simulink library "lib_all"  (Simulink 7.6)
settings: Solver=ode45  SolverMode=SingleTasking  StartTime=0.0  StopTime=10.0  RelTol=1e-3
values from neighbouring files (symbols this model uses):
  map_lib_03 = [6.8679 7.0159 7.4571 8.1849 9.1892 10.456 11.97 13.71 15.655 17.781 20.063 22.474 ... (803 elements, 73x11)]    [scr_simdata.mat]    (also in scr_simdata_all.mat)
  map_lib_06 = [2.5784 2.6727 2.9588 3.4453 4.1444 5.068 6.226 7.6242 9.2634 11.139 13.24 15.552 ... (803 elements, 73x11)]    [scr_simdata.mat]    (also in scr_simdata_all.mat)
  map_lib_09 = [0.0025784 0.0026727 0.0029588 0.0034453 0.0041444 0.005068 0.006226 0.0076242 0.0092634 0.011139 0.01324 0.015552 ... (803 elements, 73x11)]    [scr_simdata.mat]    (also in scr_simdata_all.mat)
  map_lib_10 = [2.74 2.75 2.76 2.77 2.78 2.79 2.8 2.81 2.82 2.83 2.84 2.85 ... (81 elements, 1x81)]    [scr_simdata.mat]    (also in scr_simdata_all.mat)
  map_lib_11 = [1.1983 1.2027 1.207 1.2113 1.2156 1.2198 1.2241 1.2283 1.2326 1.2368 1.241 1.2451 ... (81 elements, 1x81)]    [scr_simdata.mat]    (also in scr_simdata_all.mat)
  map_lib_12 = [2.74 2.75 2.76 2.77 2.78 2.79 2.8 2.81 2.82 2.83 2.84 2.85 ... (81 elements, 1x81)]    [scr_simdata.mat]    (also in scr_simdata_all.mat)
  map_lib_13 = [1.74 1.75 1.76 1.77 1.78 1.79 1.8 1.81 1.82 1.83 1.84 1.85 ... (81 elements, 1x81)]    [scr_simdata.mat]    (also in scr_simdata_all.mat)
  map_lib_16 = [0.00048828125 1.6159 1.8962 2.0821 2.2251 2.3426 2.4433 2.5318 2.611 2.683 2.749 2.8101 ... (1419 elements, 129x11)]    [scr_simdata.mat]    (also in scr_simdata_all.mat)
  map_mr2_01 = [0 800 1600 2400 3200 4000]    [scr_simdata.mat]    (also in scr_simdata_all.mat)
  map_mr2_02 = [1.32 1.32 1.33 1.34 1.36 1.38]    [scr_simdata.mat]    (also in scr_simdata_all.mat)
  map_mr2_03 = [0 800 1600 2400 3200 4000]    [scr_simdata.mat]    (also in scr_simdata_all.mat)
  map_mr2_04 = [1.32 1.32 1.33 1.34 1.36 1.38]    [scr_simdata.mat]    (also in scr_simdata_all.mat)
  map_mr2_05 = [0 800 1600 2400 3200 4000]    [scr_simdata.mat]    (also in scr_simdata_all.mat)
  map_mr2_06 = [1.32 1.32 1.33 1.34 1.36 1.38]    [scr_simdata.mat]    (also in scr_simdata_all.mat)
  Parameter1 = 6    [scr_simdata.mat]    (also in scr_simdata_all.mat)
  Parameter101 = 30    [scr_simdata.mat]    (also in scr_simdata_all.mat)
  Parameter102 = 12    [scr_simdata.mat]    (also in scr_simdata_all.mat)
  Parameter103 = -240    [scr_simdata.mat]    (also in scr_simdata_all.mat)
  Parameter108 = 48    [scr_simdata.mat]    (also in scr_simdata_all.mat)
  Parameter120 = 16    [scr_simdata.mat]    (also in scr_simdata_all.mat)
  Parameter121 = 87    [scr_simdata.mat]    (also in scr_simdata_all.mat)
  Parameter123 = 78    [scr_simdata.mat]    (also in scr_simdata_all.mat)
  Parameter124 = 60    [scr_simdata.mat]    (also in scr_simdata_all.mat)
  Parameter125 = -480    [scr_simdata.mat]    (also in scr_simdata_all.mat)
  Parameter130 = 96    [scr_simdata.mat]    (also in scr_simdata_all.mat)
  Parameter131 = 3    [scr_simdata.mat]    (also in scr_simdata_all.mat)
  Parameter144 = 3    [scr_simdata.mat]    (also in scr_simdata_all.mat)
  Parameter18 = 128    [scr_simdata.mat]    (also in scr_simdata_all.mat)
  Parameter19 = 16    [scr_simdata.mat]    (also in scr_simdata_all.mat)
  Parameter2 = 12    [scr_simdata.mat]    (also in scr_simdata_all.mat)
  Parameter20 = 7    [scr_simdata.mat]    (also in scr_simdata_all.mat)
  Parameter21 = 4    [scr_simdata.mat]    (also in scr_simdata_all.mat)
  Parameter3 = 18    [scr_simdata.mat]    (also in scr_simdata_all.mat)
  Parameter32 = 1    [scr_simdata.mat]    (also in scr_simdata_all.mat)
  Parameter34 = 24    [scr_simdata.mat]    (also in scr_simdata_all.mat)
  Parameter4 = 138    [scr_simdata.mat]    (also in scr_simdata_all.mat)
  Parameter42 = 48    [scr_simdata.mat]    (also in scr_simdata_all.mat)
  Parameter5 = 135    [scr_simdata.mat]    (also in scr_simdata_all.mat)
  Parameter55 = 0    [scr_simdata.mat]    (also in scr_simdata_all.mat)
  Parameter56 = 0    [scr_simdata.mat]    (also in scr_simdata_all.mat)
  Parameter57 = 1    [scr_simdata.mat]    (also in scr_simdata_all.mat)
  Parameter58 = 1    [scr_simdata.mat]    (also in scr_simdata_all.mat)
  Parameter59 = 2    [scr_simdata.mat]    (also in scr_simdata_all.mat)
  Parameter6 = 132    [scr_simdata.mat]    (also in scr_simdata_all.mat)
  Parameter60 = 2    [scr_simdata.mat]    (also in scr_simdata_all.mat)
  Parameter63 = 144    [scr_simdata.mat]    (also in scr_simdata_all.mat)
  Parameter7 = 126    [scr_simdata.mat]    (also in scr_simdata_all.mat)
  Parameter76 = 64    [scr_simdata.mat]    (also in scr_simdata_all.mat)
  Parameter77 = 135    [scr_simdata.mat]    (also in scr_simdata_all.mat)
  Parameter79 = 126    [scr_simdata.mat]    (also in scr_simdata_all.mat)
  Parameter80 = 108    [scr_simdata.mat]    (also in scr_simdata_all.mat)
  Parameter81 = 0    [scr_simdata.mat]    (also in scr_simdata_all.mat)
  Parameter86 = 0    [scr_simdata.mat]    (also in scr_simdata_all.mat)
  Parameter98 = 112    [scr_simdata.mat]    (also in scr_simdata_all.mat)
  Parameter99 = 39    [scr_simdata.mat]    (also in scr_simdata_all.mat)
undefined symbols (not defined in the file or in its folder): cylvolkp_hih_map2h, cylvolkp_map2h, pk1k_map2h
libraries: simulink x2
(part 8 of 36)

== system lib_all/SubSystem1860/SubSystem1864/SubSystem1865 ==
  note: ***編集禁止***
  In1 : Inport
  In2 : Inport  Port=2
  LO1 : Logic
  RO1 : RelationalOperator  Operator=<  InputSameDT=on
  RO2 : RelationalOperator  Operator=~=  InputSameDT=on
  single1 : DataTypeConversion  OutDataTypeStr=single  SaturateOnIntegerOverflow=on  RndMeth=Zero
  single2 : DataTypeConversion  OutDataTypeStr=single  SaturateOnIntegerOverflow=on  RndMeth=Zero
  Out1 : Outport
  wires:
  In2/1 -> single2/1
  In1/1 -> single1/1
  single1/1 -> RO1/1, RO2/1
  single2/1 -> RO1/2, RO2/2
  RO1/1 -> LO1/1
  RO2/1 -> LO1/2
  LO1/1 -> Out1/1

== system lib_all/SubSystem1860/SubSystem1864/SubSystem1866 ==
  note: ***編集禁止***
  In1 : Inport
  In2 : Inport  Port=2
  RO1 : RelationalOperator  Operator===  InputSameDT=on
  single1 : DataTypeConversion  OutDataTypeStr=single  SaturateOnIntegerOverflow=on  RndMeth=Zero
  single2 : DataTypeConversion  OutDataTypeStr=single  SaturateOnIntegerOverflow=on  RndMeth=Zero
  Out1 : Outport
  wires:
  RO1/1 -> Out1/1
  In2/1 -> single2/1
  In1/1 -> single1/1
  single1/1 -> RO1/1
  single2/1 -> RO1/2

== system lib_all/SubSystem1860/SubSystem1864/SubSystem1867 ==
  Block5123 : Inport
  Block5124 : Inport  Port=2
  Block5125 : MultiPortSwitch  zeroidx=off  RndMeth=Zero  SaturateOnIntegerOverflow=on  InputSameDT=on
  SubSystem1868 : Subsystem  TreatAsAtomicUnit=on  mask(***ATC.DSRVEC***){bd_version=1.01.A, r0=DSM7, r1=-1, r2=, r3=, r4=, r5=, r6=, r7=, r8=, r9=, c0=, c1=, c2=, c3=, c4=, c5=, c6=, c7=, c8=, c9=}
      mask prompts: bd_version "Version"
      mask help: これはAutoCからのブロックです．編集禁止．
      settings: OpenFcn=warndlg('変更禁止!');
      mask Help: web(ui_blockhelpmng(gcb));
      mask Display: disp('ATC.DSRVEC')
  SubSystem1869 : Subsystem  TreatAsAtomicUnit=on  mask(***ATC.DSRVEC***){bd_version=1.01.A, r0=DSM6, r1=-1, r2=, r3=, r4=, r5=, r6=, r7=, r8=, r9=, c0=, c1=, c2=, c3=, c4=, c5=, c6=, c7=, c8=, c9=}
      mask prompts: bd_version "Version"
      mask help: これはAutoCからのブロックです．編集禁止．
      settings: OpenFcn=warndlg('変更禁止!');
      mask Help: web(ui_blockhelpmng(gcb));
      mask Display: disp('ATC.DSRVEC')
  SubSystem1870 : Subsystem  TreatAsAtomicUnit=on  mask(***ATC.DSRVEC***){bd_version=1.01.A, r0=DSM19, r1=-1, r2=, r3=, r4=, r5=, r6=, r7=, r8=, r9=, c0=, c1=, c2=, c3=, c4=, c5=, c6=, c7=, c8=, c9=}
      mask prompts: bd_version "Version"
      mask help: これはAutoCからのブロックです．編集禁止．
      settings: OpenFcn=warndlg('変更禁止!');
      mask Help: web(ui_blockhelpmng(gcb));
      mask Display: disp('ATC.DSRVEC')
  Block5126 : Outport
  wires:
  Block5123/1 -> Block5125/1
  Block5125/1 -> Block5126/1
  SubSystem1868/1(Output) -> Block5125/2
  SubSystem1869/1(Output) -> Block5125/3
  SubSystem1870/1(Output) -> Block5125/4
  Block5124/1 -> SubSystem1868/1(element), SubSystem1869/1(element), SubSystem1870/1(element)

== system lib_all/SubSystem1879/SubSystem1881/SubSystem1882 ==
  note: ***編集禁止***
  mult : Inport
  div : Inport  Port=2
  init : Inport  Port=3
  Product : Subsystem  TreatAsAtomicUnit=on
      settings: RTWSystemCode=Inline
  Out : Outport
  wires:
  Product/1(Out) -> Out/1
  mult/1 -> Product/1(mult)
  div/1 -> Product/2(div)
  init/1 -> Product/3(init)

== system lib_all/SubSystem1879/SubSystem1881/SubSystem1883 ==
  note: ***編集禁止***
  element : Inport
  Inside_DSR : DataStoreRead  DataStoreName=DSM95
  Selector : Selector  IndexOptions=Index vector (port)  Indices=[1 3]  OutputSizes=1
  Output : Outport
  wires:
  Selector/1 -> Output/1
  element/1 -> Selector/2
  Inside_DSR/1 -> Selector/1

== system lib_all/SubSystem1879/SubSystem1881/SubSystem1884 ==
  Block5162 : Inport
  Block5163 : Inport  Port=2
  Block5164 : Inport  Port=3
  Block5165 : Sum  OutDataTypeStr=double  RndMeth=Zero  SaturateOnIntegerOverflow=on  InputSameDT=on
  Block5166 : Sum  Inputs=+-  OutDataTypeStr=double  RndMeth=Zero  SaturateOnIntegerOverflow=on  InputSameDT=on
  Block5167 : Switch  Threshold=0.5  RndMeth=Zero  SaturateOnIntegerOverflow=on  InputSameDT=on
  Block5168 : Switch  Threshold=0.5  RndMeth=Zero  SaturateOnIntegerOverflow=on  InputSameDT=on
  SubSystem1885 : Subsystem
  SubSystem1886 : Subsystem
  Block5169 : Outport
  wires:
  Block5163/1 -> SubSystem1885/1(In1), Block5166/1, SubSystem1886/1(In1), Block5168/3, Block5165/2
  Block5162/1 -> Block5165/1, Block5166/2, SubSystem1885/2(In2)
  SubSystem1885/1(Out1) -> Block5167/2
  Block5164/1 -> SubSystem1886/2(In2)
  SubSystem1886/1(Out1) -> Block5168/2
  Block5168/1 -> Block5167/3
  Block5165/1 -> Block5168/1
  Block5167/1 -> Block5169/1
  Block5166/1 -> Block5167/1

== system lib_all/SubSystem1879/SubSystem1887/SubSystem1888 ==
  note: ***編集禁止***
  In1 : Inport
  In2 : Inport  Port=2
  LO1 : Logic
  RO1 : RelationalOperator  Operator=<  InputSameDT=on
  RO2 : RelationalOperator  Operator=~=  InputSameDT=on
  single1 : DataTypeConversion  OutDataTypeStr=single  SaturateOnIntegerOverflow=on  RndMeth=Zero
  single2 : DataTypeConversion  OutDataTypeStr=single  SaturateOnIntegerOverflow=on  RndMeth=Zero
  Out1 : Outport
  wires:
  LO1/1 -> Out1/1
  RO2/1 -> LO1/2
  RO1/1 -> LO1/1
  In2/1 -> single2/1
  In1/1 -> single1/1
  single1/1 -> RO2/1, RO1/1
  single2/1 -> RO2/2, RO1/2

== system lib_all/SubSystem1879/SubSystem1887/SubSystem1889 ==
  note: ***編集禁止***
  In1 : Inport
  In2 : Inport  Port=2
  RO1 : RelationalOperator  Operator===  InputSameDT=on
  single1 : DataTypeConversion  OutDataTypeStr=single  SaturateOnIntegerOverflow=on  RndMeth=Zero
  single2 : DataTypeConversion  OutDataTypeStr=single  SaturateOnIntegerOverflow=on  RndMeth=Zero
  Out1 : Outport
  wires:
  RO1/1 -> Out1/1
  In2/1 -> single2/1
  In1/1 -> single1/1
  single1/1 -> RO1/1
  single2/1 -> RO1/2

== system lib_all/SubSystem1879/SubSystem1887/SubSystem1890 ==
  note: ***編集禁止***
  In1 : Inport
  In2 : Inport  Port=2
  RO1 : RelationalOperator  Operator===  InputSameDT=on
  single1 : DataTypeConversion  OutDataTypeStr=single  SaturateOnIntegerOverflow=on  RndMeth=Zero
  single2 : DataTypeConversion  OutDataTypeStr=single  SaturateOnIntegerOverflow=on  RndMeth=Zero
  Out1 : Outport
  wires:
  RO1/1 -> Out1/1
  In2/1 -> single2/1
  In1/1 -> single1/1
  single1/1 -> RO1/1
  single2/1 -> RO1/2

== system lib_all/SubSystem1879/SubSystem1887/SubSystem1891 ==
  Block5190 : Inport
  Block5191 : Inport  Port=2
  Block5192 : EnablePort
  Block5193 : Demux  Outputs=2
  Block5194 : Mux  Inputs=2
  SubSystem1892 : Subsystem
  SubSystem1896 : Subsystem
  Block5203 : Outport
  wires:
  Block5190/1 -> Block5193/1
  Block5191/1 -> SubSystem1896/1(Block5199), SubSystem1892/1(Block5195)
  Block5193/1 -> SubSystem1892/2(Block5196)
  Block5193/2 -> SubSystem1896/2(Block5200)
  SubSystem1892/1(Block5198) -> Block5194/1
  SubSystem1896/1(Block5202) -> Block5194/2
  Block5194/1 -> Block5203/1

== system lib_all/SubSystem1879/SubSystem1887/SubSystem1900 ==
  Block5204 : Inport
  Block5205 : Inport  Port=2
  Block5206 : EnablePort
  Block5207 : Demux  Outputs=2
  Block5208 : Mux  Inputs=2
  Block5209 : Sum  OutDataTypeStr=double  RndMeth=Zero  SaturateOnIntegerOverflow=on  InputSameDT=on
  SubSystem1901 : Subsystem  TreatAsAtomicUnit=on  mask(***ATC.DSRVEC***){bd_version=1.01.A, r0=DSM4, r1=-1, r2=, r3=, r4=, r5=, r6=, r7=, r8=, r9=, c0=, c1=, c2=, c3=, c4=, c5=, c6=, c7=, c8=, c9=}
      mask prompts: bd_version "Version"
      mask help: これはAutoCからのブロックです．編集禁止．
      settings: OpenFcn=warndlg('変更禁止!');
      mask Help: web(ui_blockhelpmng(gcb));
      mask Display: disp('ATC.DSRVEC')
  SubSystem1902 : Subsystem
  SubSystem1906 : Subsystem
  Block5218 : Outport
  wires:
  Block5207/2 -> SubSystem1906/2(Block5215)
  Block5207/1 -> SubSystem1902/2(Block5211)
  Block5204/1 -> Block5207/1
  SubSystem1902/1(Block5213) -> Block5208/1
  SubSystem1906/1(Block5217) -> Block5208/2
  Block5209/1 -> Block5218/1
  SubSystem1901/1(Output) -> Block5209/1
  Block5208/1 -> Block5209/2
  Block5205/1 -> SubSystem1906/1(Block5214), SubSystem1902/1(Block5210), SubSystem1901/1(element)

== system lib_all/SubSystem1879/SubSystem1887/SubSystem1910 ==
  Block5219 : Inport
  Block5220 : Inport  Port=2
  Block5221 : EnablePort
  Block5222 : Demux  Outputs=2
  Block5223 : Mux  Inputs=2
  Block5224 : Sum  OutDataTypeStr=double  RndMeth=Zero  SaturateOnIntegerOverflow=on  InputSameDT=on
  SubSystem1911 : Subsystem  TreatAsAtomicUnit=on  mask(***ATC.DSRVEC***){bd_version=1.01.A, r0=DSM2, r1=-1, r2=, r3=, r4=, r5=, r6=, r7=, r8=, r9=, c0=, c1=, c2=, c3=, c4=, c5=, c6=, c7=, c8=, c9=}
      mask prompts: bd_version "Version"
      mask help: これはAutoCからのブロックです．編集禁止．
      settings: OpenFcn=warndlg('変更禁止!');
      mask Help: web(ui_blockhelpmng(gcb));
      mask Display: disp('ATC.DSRVEC')
  SubSystem1912 : Subsystem
  SubSystem1916 : Subsystem
  Block5233 : Outport
  wires:
  Block5219/1 -> Block5222/1
  Block5222/1 -> SubSystem1912/2(Block5226)
  Block5222/2 -> SubSystem1916/2(Block5230)
  SubSystem1912/1(Block5228) -> Block5223/1
  SubSystem1916/1(Block5232) -> Block5223/2
  Block5224/1 -> Block5233/1
  Block5223/1 -> Block5224/2
  Block5220/1 -> SubSystem1916/1(Block5229), SubSystem1912/1(Block5225), SubSystem1911/1(element)
  SubSystem1911/1(Output) -> Block5224/1

== system lib_all/SubSystem1920/SubSystem1922/SubSystem1923 ==
  note: ***編集禁止***
  mult : Inport
  div : Inport  Port=2
  init : Inport  Port=3
  Product : Subsystem  TreatAsAtomicUnit=on
      settings: RTWSystemCode=Inline
  Out : Outport
  wires:
  init/1 -> Product/3(init)
  div/1 -> Product/2(div)
  mult/1 -> Product/1(mult)
  Product/1(Out) -> Out/1

== system lib_all/SubSystem1920/SubSystem1922/SubSystem1924 ==
  note: ***編集禁止***
  element : Inport
  Inside_DSR : DataStoreRead  DataStoreName=DSM95
  Selector : Selector  IndexOptions=Index vector (port)  Indices=[1 3]  OutputSizes=1
  Output : Outport
  wires:
  Inside_DSR/1 -> Selector/1
  element/1 -> Selector/2
  Selector/1 -> Output/1

== system lib_all/SubSystem1920/SubSystem1922/SubSystem1925 ==
  Block5262 : Inport
  Block5263 : Inport  Port=2
  Block5264 : Inport  Port=3
  Block5265 : Sum  OutDataTypeStr=double  RndMeth=Zero  SaturateOnIntegerOverflow=on  InputSameDT=on
  Block5266 : Sum  Inputs=+-  OutDataTypeStr=double  RndMeth=Zero  SaturateOnIntegerOverflow=on  InputSameDT=on
  Block5267 : Switch  Threshold=0.5  RndMeth=Zero  SaturateOnIntegerOverflow=on  InputSameDT=on
  Block5268 : Switch  Threshold=0.5  RndMeth=Zero  SaturateOnIntegerOverflow=on  InputSameDT=on
  SubSystem1926 : Subsystem
  SubSystem1927 : Subsystem
  Block5269 : Outport
  wires:
  Block5266/1 -> Block5267/1
  Block5267/1 -> Block5269/1
  Block5265/1 -> Block5268/1
  Block5268/1 -> Block5267/3
  SubSystem1927/1(Out1) -> Block5268/2
  Block5264/1 -> SubSystem1927/2(In2)
  SubSystem1926/1(Out1) -> Block5267/2
  Block5262/1 -> SubSystem1926/2(In2), Block5266/2, Block5265/1
  Block5263/1 -> Block5265/2, Block5268/3, SubSystem1927/1(In1), Block5266/1, SubSystem1926/1(In1)

== system lib_all/SubSystem1920/SubSystem1928/SubSystem1929 ==
  note: ***編集禁止***
  In1 : Inport
  In2 : Inport  Port=2
  LO1 : Logic
  RO1 : RelationalOperator  Operator=<  InputSameDT=on
  RO2 : RelationalOperator  Operator=~=  InputSameDT=on
  single1 : DataTypeConversion  OutDataTypeStr=single  SaturateOnIntegerOverflow=on  RndMeth=Zero
  single2 : DataTypeConversion  OutDataTypeStr=single  SaturateOnIntegerOverflow=on  RndMeth=Zero
  Out1 : Outport
  wires:
  single2/1 -> RO1/2, RO2/2
  single1/1 -> RO1/1, RO2/1
  In1/1 -> single1/1
  In2/1 -> single2/1
  RO1/1 -> LO1/1
  RO2/1 -> LO1/2
  LO1/1 -> Out1/1

== system lib_all/SubSystem1920/SubSystem1928/SubSystem1930 ==
  note: ***編集禁止***
  In1 : Inport
  In2 : Inport  Port=2
  RO1 : RelationalOperator  Operator===  InputSameDT=on
  single1 : DataTypeConversion  OutDataTypeStr=single  SaturateOnIntegerOverflow=on  RndMeth=Zero
  single2 : DataTypeConversion  OutDataTypeStr=single  SaturateOnIntegerOverflow=on  RndMeth=Zero
  Out1 : Outport
  wires:
  single2/1 -> RO1/2
  single1/1 -> RO1/1
  In1/1 -> single1/1
  In2/1 -> single2/1
  RO1/1 -> Out1/1

== system lib_all/SubSystem1920/SubSystem1928/SubSystem1931 ==
  note: ***編集禁止***
  In1 : Inport
  In2 : Inport  Port=2
  RO1 : RelationalOperator  Operator===  InputSameDT=on
  single1 : DataTypeConversion  OutDataTypeStr=single  SaturateOnIntegerOverflow=on  RndMeth=Zero
  single2 : DataTypeConversion  OutDataTypeStr=single  SaturateOnIntegerOverflow=on  RndMeth=Zero
  Out1 : Outport
  wires:
  single2/1 -> RO1/2
  single1/1 -> RO1/1
  In1/1 -> single1/1
  In2/1 -> single2/1
  RO1/1 -> Out1/1

== system lib_all/SubSystem1920/SubSystem1928/SubSystem1932 ==
  Block5290 : Inport
  Block5291 : Inport  Port=2
  Block5292 : EnablePort
  Block5293 : Demux  Outputs=2
  Block5294 : Mux  Inputs=2
  SubSystem1933 : Subsystem
  SubSystem1937 : Subsystem
  Block5303 : Outport
  wires:
  Block5294/1 -> Block5303/1
  SubSystem1937/1(Block5302) -> Block5294/2
  SubSystem1933/1(Block5298) -> Block5294/1
  Block5293/2 -> SubSystem1937/2(Block5300)
  Block5293/1 -> SubSystem1933/2(Block5296)
  Block5291/1 -> SubSystem1933/1(Block5295), SubSystem1937/1(Block5299)
  Block5290/1 -> Block5293/1

== system lib_all/SubSystem1920/SubSystem1928/SubSystem1941 ==
  Block5304 : Inport
  Block5305 : Inport  Port=2
  Block5306 : EnablePort
  Block5307 : Demux  Outputs=2
  Block5308 : Mux  Inputs=2
  Block5309 : Sum  OutDataTypeStr=double  RndMeth=Zero  SaturateOnIntegerOverflow=on  InputSameDT=on
  SubSystem1942 : Subsystem  TreatAsAtomicUnit=on  mask(***ATC.DSRVEC***){bd_version=1.01.A, r0=DSM4, r1=-1, r2=, r3=, r4=, r5=, r6=, r7=, r8=, r9=, c0=, c1=, c2=, c3=, c4=, c5=, c6=, c7=, c8=, c9=}
      mask prompts: bd_version "Version"
      mask help: これはAutoCからのブロックです．編集禁止．
      settings: OpenFcn=warndlg('変更禁止!');
      mask Help: web(ui_blockhelpmng(gcb));
      mask Display: disp('ATC.DSRVEC')
  SubSystem1943 : Subsystem
  SubSystem1947 : Subsystem
  Block5318 : Outport
  wires:
  Block5305/1 -> SubSystem1942/1(element), SubSystem1943/1(Block5310), SubSystem1947/1(Block5314)
  Block5308/1 -> Block5309/2
  SubSystem1942/1(Output) -> Block5309/1
  Block5309/1 -> Block5318/1
  SubSystem1947/1(Block5317) -> Block5308/2
  SubSystem1943/1(Block5313) -> Block5308/1
  Block5304/1 -> Block5307/1
  Block5307/1 -> SubSystem1943/2(Block5311)
  Block5307/2 -> SubSystem1947/2(Block5315)

== system lib_all/SubSystem1920/SubSystem1928/SubSystem1951 ==
  Block5319 : Inport
  Block5320 : Inport  Port=2
  Block5321 : EnablePort
  Block5322 : Demux  Outputs=2
  Block5323 : Mux  Inputs=2
  Block5324 : Sum  OutDataTypeStr=double  RndMeth=Zero  SaturateOnIntegerOverflow=on  InputSameDT=on
  SubSystem1952 : Subsystem  TreatAsAtomicUnit=on  mask(***ATC.DSRVEC***){bd_version=1.01.A, r0=DSM2, r1=-1, r2=, r3=, r4=, r5=, r6=, r7=, r8=, r9=, c0=, c1=, c2=, c3=, c4=, c5=, c6=, c7=, c8=, c9=}
      mask prompts: bd_version "Version"
      mask help: これはAutoCからのブロックです．編集禁止．
      settings: OpenFcn=warndlg('変更禁止!');
      mask Help: web(ui_blockhelpmng(gcb));
      mask Display: disp('ATC.DSRVEC')
  SubSystem1953 : Subsystem
  SubSystem1957 : Subsystem
  Block5333 : Outport
  wires:
  SubSystem1952/1(Output) -> Block5324/1
  Block5320/1 -> SubSystem1952/1(element), SubSystem1953/1(Block5325), SubSystem1957/1(Block5329)
  Block5323/1 -> Block5324/2
  Block5324/1 -> Block5333/1
  SubSystem1957/1(Block5332) -> Block5323/2
  SubSystem1953/1(Block5328) -> Block5323/1
  Block5322/2 -> SubSystem1957/2(Block5330)
  Block5322/1 -> SubSystem1953/2(Block5326)
  Block5319/1 -> Block5322/1

== system lib_all/SubSystem347/SubSystem349/SubSystem350 ==
  note: ***編集禁止***
  In1 : Inport
  In2 : Inport  Port=2
  LO1 : Logic
  RO1 : RelationalOperator  Operator=<  InputSameDT=on
  RO2 : RelationalOperator  Operator=~=  InputSameDT=on
  single1 : DataTypeConversion  OutDataTypeStr=single  SaturateOnIntegerOverflow=on  RndMeth=Zero
  single2 : DataTypeConversion  OutDataTypeStr=single  SaturateOnIntegerOverflow=on  RndMeth=Zero
  Out1 : Outport
  wires:
  In2/1 -> single2/1
  In1/1 -> single1/1
  single1/1 -> RO1/1, RO2/1
  single2/1 -> RO1/2, RO2/2
  RO1/1 -> LO1/1
  RO2/1 -> LO1/2
  LO1/1 -> Out1/1

== system lib_all/SubSystem347/SubSystem349/SubSystem351 ==
  note: ***編集禁止***
  element : Inport
  Inside_DSR : DataStoreRead  DataStoreName=DSM386
  Selector : Selector  IndexOptions=Index vector (port)  Indices=[1 3]  OutputSizes=1
  Output : Outport
  wires:
  Selector/1 -> Output/1
  element/1 -> Selector/2
  Inside_DSR/1 -> Selector/1

== system lib_all/SubSystem347/SubSystem349/SubSystem352 ==
  Block767 : Inport
  Block768 : Inport  Port=2
  Block769 : Inport  Port=3
  Block770 : Inport  Port=4
  Block771 : EnablePort
  Block772 : Constant  OutDataTypeStr=double  SampleTime=-1
  Block773 : Constant  Value=0  OutDataTypeStr=double  SampleTime=-1
  Block774 : Constant  Value=0  OutDataTypeStr=double  SampleTime=-1
  Block775 : Sum  OutDataTypeStr=double  RndMeth=Zero  SaturateOnIntegerOverflow=on  InputSameDT=on
  SubSystem353 : Subsystem  TreatAsAtomicUnit=on
      settings: AttributesFormatString=Attribute171  RTWFcnNameOpts=Use subsystem name
  SubSystem406 : Subsystem  TreatAsAtomicUnit=on
      settings: AttributesFormatString=Attribute190  RTWFcnNameOpts=Use subsystem name
  SubSystem459 : Subsystem
  SubSystem466 : Subsystem
  SubSystem473 : Subsystem  Priority=3  TreatAsAtomicUnit=on
      settings: AttributesFormatString=Attribute209  RTWSystemCode=Inline
  wires:
  Block772/1 -> Block775/2
  Block767/1 -> SubSystem406/1(Block898), SubSystem353/1(Block776), SubSystem473/1(Block1048), Block775/1
  Block769/1 -> SubSystem353/2(Block777)
  Block768/1 -> SubSystem406/2(Block899)
  Block774/1 -> SubSystem353/4(Block779), SubSystem353/3(Block778)
  Block773/1 -> SubSystem406/4(Block901), SubSystem406/3(Block900)
  SubSystem353/1(Block897) -> SubSystem466/1(Block1034)
  Block770/1 -> SubSystem466/3(Block1036), SubSystem459/3(Block1022)
  Block775/1 -> SubSystem466/2(Block1035), SubSystem459/2(Block1021)
  SubSystem406/1(Block1019) -> SubSystem459/1(Block1020)

== system lib_all/SubSystem347/SubSystem349/SubSystem476 ==
  Block1053 : Inport
  Block1054 : Constant  Value=Parameter99  OutDataTypeStr=double  SampleTime=-1
  Block1055 : Constant  Value=Parameter121  OutDataTypeStr=double  SampleTime=-1
  Block1056 : Constant  Value=Parameter79  OutDataTypeStr=double  SampleTime=-1
  Block1057 : Constant  Value=Parameter77  OutDataTypeStr=double  SampleTime=-1
  Block1058 : Constant  Value=Parameter101  OutDataTypeStr=double  SampleTime=-1
  Block1059 : Constant  Value=Parameter123  OutDataTypeStr=double  SampleTime=-1
  Block1060 : MultiPortSwitch  zeroidx=off  RndMeth=Zero  SaturateOnIntegerOverflow=on  InputSameDT=on
  Block1061 : Mux  Inputs=2
  Block1062 : Mux  Inputs=2
  Block1063 : Mux  Inputs=2
  Block1064 : Outport
  wires:
  Block1056/1 -> Block1061/2
  Block1058/1 -> Block1062/2
  Block1059/1 -> Block1063/2
  Block1055/1 -> Block1063/1
  Block1054/1 -> Block1062/1
  Block1060/1 -> Block1064/1
  Block1053/1 -> Block1060/1
  Block1057/1 -> Block1061/1
  Block1061/1 -> Block1060/2
  Block1063/1 -> Block1060/4
  Block1062/1 -> Block1060/3

== system lib_all/SubSystem347/SubSystem477/SubSystem478 ==
  note: ***編集禁止***
  In1 : Inport
  In2 : Inport  Port=2
  RO1 : RelationalOperator  Operator===  InputSameDT=on
  single1 : DataTypeConversion  OutDataTypeStr=single  SaturateOnIntegerOverflow=on  RndMeth=Zero
  single2 : DataTypeConversion  OutDataTypeStr=single  SaturateOnIntegerOverflow=on  RndMeth=Zero
  Out1 : Outport
  wires:
  RO1/1 -> Out1/1
  In2/1 -> single2/1
  In1/1 -> single1/1
  single1/1 -> RO1/1
  single2/1 -> RO1/2

== system lib_all/SubSystem347/SubSystem477/SubSystem479 ==
  Block1069 : Inport
  Block1070 : EnablePort
  Block1071 : Constant  Value=Parameter18  OutDataTypeStr=double  SampleTime=-1
  SubSystem480 : Subsystem  TreatAsAtomicUnit=on
      settings: RTWSystemCode=Inline
  SubSystem482 : Subsystem
  SubSystem492 : Subsystem  TreatAsAtomicUnit=on
  wires:
  Block1069/1 -> SubSystem492/2(Block1096), SubSystem482/3(Block1077)
  Block1071/1 -> SubSystem480/1(N)
  SubSystem480/1(action) -> SubSystem492/Trigger
  SubSystem480/2(i) -> SubSystem492/1(Block1095)
  SubSystem492/1(Block1113) -> SubSystem482/1(Block1075)
  SubSystem492/2(Block1114) -> SubSystem482/2(Block1076)

== system lib_all/SubSystem347/SubSystem477/SubSystem502 ==
  note: ***編集禁止***
  element : Inport
  Inside_DSR : DataStoreRead  DataStoreName=DSM386
  Selector : Selector  IndexOptions=Index vector (port)  Indices=[1 3]  OutputSizes=1
  Output : Outport
  wires:
  Selector/1 -> Output/1
  element/1 -> Selector/2
  Inside_DSR/1 -> Selector/1

== system lib_all/SubSystem347/SubSystem503/SubSystem504 ==
  note: ***編集禁止***
  In1 : Inport
  In2 : Inport  Port=2
  LO1 : Logic  Operator=OR
  RO1 : RelationalOperator  InputSameDT=on
  RO2 : RelationalOperator  Operator===  InputSameDT=on
  single1 : DataTypeConversion  OutDataTypeStr=single  SaturateOnIntegerOverflow=on  RndMeth=Zero
  single2 : DataTypeConversion  OutDataTypeStr=single  SaturateOnIntegerOverflow=on  RndMeth=Zero
  Out1 : Outport
  wires:
  In2/1 -> single2/1
  In1/1 -> single1/1
  single1/1 -> RO1/1, RO2/1
  single2/1 -> RO1/2, RO2/2
  RO1/1 -> LO1/1
  RO2/1 -> LO1/2
  LO1/1 -> Out1/1

== system lib_all/SubSystem347/SubSystem503/SubSystem505 ==
  note: ***編集禁止***
  In1 : Inport
  In2 : Inport  Port=2
  RO1 : RelationalOperator  Operator===  InputSameDT=on
  single1 : DataTypeConversion  OutDataTypeStr=single  SaturateOnIntegerOverflow=on  RndMeth=Zero
  single2 : DataTypeConversion  OutDataTypeStr=single  SaturateOnIntegerOverflow=on  RndMeth=Zero
  Out1 : Outport
  wires:
  RO1/1 -> Out1/1
  In2/1 -> single2/1
  In1/1 -> single1/1
  single1/1 -> RO1/1
  single2/1 -> RO1/2

== system lib_all/SubSystem347/SubSystem503/SubSystem506 ==
  Block1119 : EnablePort
  Block1120 : Constant  Value=Parameter131  OutDataTypeStr=double  SampleTime=-1
  SubSystem507 : Subsystem  TreatAsAtomicUnit=on
      settings: RTWSystemCode=Inline
  SubSystem509 : Subsystem  TreatAsAtomicUnit=on
      settings: RTWSystemCode=Inline
  SubSystem511 : Subsystem  TreatAsAtomicUnit=on
  SubSystem515 : Subsystem  TreatAsAtomicUnit=on
  SubSystem517 : Subsystem  Priority=2
      settings: AttributesFormatString=Attribute243
  wires:
  Block1120/1 -> SubSystem507/1(N), SubSystem509/1(N)
  SubSystem507/1(action) -> SubSystem511/Trigger
  SubSystem507/2(i) -> SubSystem511/1(Block1127)
  SubSystem509/1(action) -> SubSystem515/Trigger
  SubSystem509/2(i) -> SubSystem515/1(Block1131)

== system lib_all/SubSystem518/SubSystem520/SubSystem521 ==
  Block1142 : Inport
  Block1143 : Constant  Value=Parameter99  OutDataTypeStr=double  SampleTime=-1
  Block1144 : Constant  Value=Parameter121  OutDataTypeStr=double  SampleTime=-1
  Block1145 : Constant  Value=Parameter79  OutDataTypeStr=double  SampleTime=-1
  Block1146 : Constant  Value=Parameter77  OutDataTypeStr=double  SampleTime=-1
  Block1147 : Constant  Value=Parameter101  OutDataTypeStr=double  SampleTime=-1
  Block1148 : Constant  Value=Parameter123  OutDataTypeStr=double  SampleTime=-1
  Block1149 : MultiPortSwitch  zeroidx=off  RndMeth=Zero  SaturateOnIntegerOverflow=on  InputSameDT=on
  Block1150 : Mux  Inputs=2
  Block1151 : Mux  Inputs=2
  Block1152 : Mux  Inputs=2
  Block1153 : Outport
  wires:
  Block1148/1 -> Block1152/2
  Block1144/1 -> Block1152/1
  Block1147/1 -> Block1151/2
  Block1143/1 -> Block1151/1
  Block1145/1 -> Block1150/2
  Block1142/1 -> Block1149/1
  Block1146/1 -> Block1150/1
  Block1150/1 -> Block1149/2
  Block1152/1 -> Block1149/4
  Block1149/1 -> Block1153/1  "SigLabel25"
  Block1151/1 -> Block1149/3

== system lib_all/SubSystem518/SubSystem520/SubSystem522 ==
  note: ***編集禁止***
  element : Inport
  Inside_DSR : DataStoreRead  DataStoreName=DSM36
  Selector : Selector  IndexOptions=Index vector (port)  Indices=[1 3]  OutputSizes=1
  Output : Outport
  wires:
  Selector/1 -> Output/1
  element/1 -> Selector/2
  Inside_DSR/1 -> Selector/1

== system lib_all/SubSystem518/SubSystem523/SubSystem524 ==
  note: Text80
  Block1163 : Inport
  Block1164 : Inport  Port=2
  Block1165 : Inport  Port=3
  Block1166 : Inport  Port=4
  Block1167 : Constant  Value=0  OutDataTypeStr=double  SampleTime=-1
  Block1168 : Constant  OutDataTypeStr=double  SampleTime=-1
  Block1169 : Constant  Value=0  OutDataTypeStr=double  SampleTime=-1
  Block1170 : Gain  Gain=1/5  SaturateOnIntegerOverflow=on  OutDataTypeStr=Inherit: Same as input  ParamDataTypeStr=Inherit: Same as input
      settings: AttributesFormatString=Attribute260
  Block1171 : Product  OutDataTypeStr=double  SaturateOnIntegerOverflow=on  RndMeth=Zero  InputSameDT=on
  Block1172 : Sum  Inputs=-+  OutDataTypeStr=double  RndMeth=Zero  SaturateOnIntegerOverflow=on  InputSameDT=on
  Block1173 : Sum  OutDataTypeStr=double  RndMeth=Zero  SaturateOnIntegerOverflow=on  InputSameDT=on
  Block1174 : Sum  OutDataTypeStr=double  RndMeth=Zero  SaturateOnIntegerOverflow=on  InputSameDT=on
  Block1175 : Switch  Threshold=0.5  RndMeth=Zero  SaturateOnIntegerOverflow=on  InputSameDT=on
  SubSystem526 : Subsystem
  SubSystem527 : Subsystem
  SubSystem533 : Subsystem
  Block1284 : Outport
  wires:
  Block1164/1 -> SubSystem527/1(Block1176)
  SubSystem527/1(Block1201) -> SubSystem533/1(Block1203)
  Block1166/1 -> SubSystem533/2(Block1204)
  SubSystem533/1(Block1282) -> Block1173/1, Block1172/1
  SubSystem533/2(Block1283) -> Block1172/2
  Block1172/1 -> Block1171/1  "SigLabel28"
  SubSystem527/2(Block1202) -> Block1171/2
  Block1173/1 -> Block1175/3, SubSystem526/1(In1)
  Block1165/1 -> SubSystem527/3(Block1178)
  Block1169/1 -> SubSystem526/2(In2)
  SubSystem526/1(Out1) -> Block1175/2
  Block1175/1 -> Block1284/1
  Block1167/1 -> Block1175/1
  Block1168/1 -> Block1174/2
  Block1171/1 -> Block1170/1
  Block1170/1 -> Block1173/2
  Block1163/1 -> Block1174/1
  Block1174/1 -> SubSystem527/2(Block1177), SubSystem533/3(Block1205)

== system lib_all/SubSystem518/SubSystem523/SubSystem577 ==
  note: Text86
  Block1285 : Inport
  Block1286 : Inport  Port=2
  Block1287 : Inport  Port=3
  Block1288 : Inport  Port=4
  Block1289 : Constant  Value=0  OutDataTypeStr=double  SampleTime=-1
  Block1290 : Constant  OutDataTypeStr=double  SampleTime=-1
  Block1291 : Constant  Value=0  OutDataTypeStr=double  SampleTime=-1
  Block1292 : Gain  Gain=1/5  SaturateOnIntegerOverflow=on  OutDataTypeStr=Inherit: Same as input  ParamDataTypeStr=Inherit: Same as input
      settings: AttributesFormatString=Attribute278
  Block1293 : Product  OutDataTypeStr=double  SaturateOnIntegerOverflow=on  RndMeth=Zero  InputSameDT=on
  Block1294 : Sum  Inputs=-+  OutDataTypeStr=double  RndMeth=Zero  SaturateOnIntegerOverflow=on  InputSameDT=on
  Block1295 : Sum  OutDataTypeStr=double  RndMeth=Zero  SaturateOnIntegerOverflow=on  InputSameDT=on
  Block1296 : Sum  OutDataTypeStr=double  RndMeth=Zero  SaturateOnIntegerOverflow=on  InputSameDT=on
  Block1297 : Switch  Threshold=0.5  RndMeth=Zero  SaturateOnIntegerOverflow=on  InputSameDT=on
  SubSystem579 : Subsystem
  SubSystem580 : Subsystem
  SubSystem586 : Subsystem
  Block1406 : Outport
  wires:
  Block1286/1 -> SubSystem580/1(Block1298)
  SubSystem580/1(Block1323) -> SubSystem586/1(Block1325)
  Block1288/1 -> SubSystem586/2(Block1326)
  SubSystem586/1(Block1404) -> Block1295/1, Block1294/1
  SubSystem586/2(Block1405) -> Block1294/2
  Block1294/1 -> Block1293/1  "SigLabel31"
  SubSystem580/2(Block1324) -> Block1293/2
  Block1295/1 -> Block1297/3, SubSystem579/1(In1)
  Block1287/1 -> SubSystem580/3(Block1300)
  Block1291/1 -> SubSystem579/2(In2)
  SubSystem579/1(Out1) -> Block1297/2
  Block1297/1 -> Block1406/1
  Block1289/1 -> Block1297/1
  Block1290/1 -> Block1296/2
  Block1293/1 -> Block1292/1
  Block1292/1 -> Block1295/2
  Block1285/1 -> Block1296/1
  Block1296/1 -> SubSystem580/2(Block1299), SubSystem586/3(Block1327)

== system lib_all/SubSystem518/SubSystem630/SubSystem631 ==
  note: ***編集禁止***
  mult : Inport
  div : Inport  Port=2
  Product : Product  Inputs=*/  SaturateOnIntegerOverflow=on  RndMeth=Zero  InputSameDT=on  OutDataTypeStr=Inherit: Same as first input
  Out : Outport
  wires:
  div/1 -> Product/2
  mult/1 -> Product/1
  Product/1 -> Out/1

== system lib_all/SubSystem518/SubSystem630/SubSystem632 ==
  note: ***編集禁止***
  In1 : Inport
  In2 : Inport  Port=2
  RO1 : RelationalOperator  Operator=<=  InputSameDT=on
  single1 : DataTypeConversion  OutDataTypeStr=single  SaturateOnIntegerOverflow=on  RndMeth=Zero
  single2 : DataTypeConversion  OutDataTypeStr=single  SaturateOnIntegerOverflow=on  RndMeth=Zero
  Out1 : Outport
  wires:
  RO1/1 -> Out1/1
  single2/1 -> RO1/2
  single1/1 -> RO1/1
  In1/1 -> single1/1
  In2/1 -> single2/1

== system lib_all/SubSystem518/SubSystem630/SubSystem633 ==
  note: ***編集禁止***
  input : Inport
  element : Inport  Port=2
  Assignment : Assignment  IndexOptions=Index vector (port)  Indices=1  OutputSizes=[1 1]
  Inside_DSR : DataStoreRead  DataStoreName=DSM45
  Inside_DSW : DataStoreWrite  DataStoreName=DSM45
  wires:
  element/1 -> Assignment/3
  input/1 -> Assignment/2
  Inside_DSR/1 -> Assignment/1
  Assignment/1 -> Inside_DSW/1

== system lib_all/SubSystem518/SubSystem630/SubSystem634 ==
  note: ***編集禁止***
  input : Inport
  element : Inport  Port=2
  Assignment : Assignment  IndexOptions=Index vector (port)  Indices=1  OutputSizes=[1 1]
  Inside_DSR : DataStoreRead  DataStoreName=DSM46
  Inside_DSW : DataStoreWrite  DataStoreName=DSM46
  wires:
  element/1 -> Assignment/3
  input/1 -> Assignment/2
  Inside_DSR/1 -> Assignment/1
  Assignment/1 -> Inside_DSW/1

== system lib_all/SubSystem518/SubSystem630/SubSystem635 ==
  note: ***編集禁止***
  input : Inport
  element : Inport  Port=2
  Assignment : Assignment  IndexOptions=Index vector (port)  Indices=1  OutputSizes=[1 1]
  Inside_DSR : DataStoreRead  DataStoreName=DSM82
  Inside_DSW : DataStoreWrite  DataStoreName=DSM82
  wires:
  element/1 -> Assignment/3
  input/1 -> Assignment/2
  Inside_DSR/1 -> Assignment/1
  Assignment/1 -> Inside_DSW/1

== system lib_all/SubSystem518/SubSystem630/SubSystem636 ==
  note: ***編集禁止***
  input : Inport
  element : Inport  Port=2
  Assignment : Assignment  IndexOptions=Index vector (port)  Indices=1  OutputSizes=[1 1]
  Inside_DSR : DataStoreRead  DataStoreName=DSM83
  Inside_DSW : DataStoreWrite  DataStoreName=DSM83
  wires:
  element/1 -> Assignment/3
  input/1 -> Assignment/2
  Inside_DSR/1 -> Assignment/1
  Assignment/1 -> Inside_DSW/1

== system lib_all/SubSystem518/SubSystem630/SubSystem637 ==
  note: ***編集禁止***
  element : Inport
  Inside_DSR : DataStoreRead  DataStoreName=DSM84
  Selector : Selector  IndexOptions=Index vector (port)  Indices=[1 3]  OutputSizes=1
  Output : Outport
  wires:
  Selector/1 -> Output/1
  element/1 -> Selector/2
  Inside_DSR/1 -> Selector/1

== system lib_all/SubSystem518/SubSystem630/SubSystem638 ==
  note: ***編集禁止***
  input : Inport
  element : Inport  Port=2
  Assignment : Assignment  IndexOptions=Index vector (port)  Indices=1  OutputSizes=[1 1]
  Inside_DSR : DataStoreRead  DataStoreName=DSM84
  Inside_DSW : DataStoreWrite  DataStoreName=DSM84
  wires:
  element/1 -> Assignment/3
  input/1 -> Assignment/2
  Inside_DSR/1 -> Assignment/1
  Assignment/1 -> Inside_DSW/1

== system lib_all/SubSystem518/SubSystem630/SubSystem639 ==
  note: ***編集禁止***
  element : Inport
  Inside_DSR : DataStoreRead  DataStoreName=DSM9
  Selector : Selector  IndexOptions=Index vector (port)  Indices=[1 3]  OutputSizes=1
  Output : Outport
  wires:
  Selector/1 -> Output/1
  element/1 -> Selector/2
  Inside_DSR/1 -> Selector/1

== system lib_all/SubSystem518/SubSystem630/SubSystem640 ==
  note: ***編集禁止***
  input : Inport
  element : Inport  Port=2
  Assignment : Assignment  IndexOptions=Index vector (port)  Indices=1  OutputSizes=[1 1]
  Inside_DSR : DataStoreRead  DataStoreName=DSM9
  Inside_DSW : DataStoreWrite  DataStoreName=DSM9
  wires:
  element/1 -> Assignment/3
  input/1 -> Assignment/2
  Inside_DSR/1 -> Assignment/1
  Assignment/1 -> Inside_DSW/1

== system lib_all/SubSystem518/SubSystem630/SubSystem641 ==
  note: Text87
  Block1426 : Inport
  Block1427 : Inport  Port=2
  Block1428 : Inport  Port=3
  Block1429 : Constant  OutDataTypeStr=double  SampleTime=-1
  Block1430 : MinMax  Function=max  Inputs=2  RndMeth=Zero  SaturateOnIntegerOverflow=on  InputSameDT=on
  Block1431 : Product  Inputs=*/  OutDataTypeStr=double  SaturateOnIntegerOverflow=on  RndMeth=Zero  InputSameDT=on
      settings: AttributesFormatString=Attribute298
  Block1432 : Sum  Inputs=+-  OutDataTypeStr=double  RndMeth=Zero  SaturateOnIntegerOverflow=on  InputSameDT=on
  Block1433 : Sum  Inputs=2  OutDataTypeStr=double  RndMeth=Zero  SaturateOnIntegerOverflow=on  InputSameDT=on
  Block1434 : Outport
  wires:
  Block1430/1 -> Block1431/2
  Block1428/1 -> Block1430/1
  Block1429/1 -> Block1430/2
  Block1433/1 -> Block1434/1
  Block1431/1 -> Block1433/1
  Block1432/1 -> Block1431/1
  Block1427/1 -> Block1432/1
  Block1426/1 -> Block1432/2, Block1433/2

== system lib_all/SubSystem518/SubSystem630/SubSystem642 ==
  note: Text88
  Block1435 : Inport
  Block1436 : Inport  Port=2
  Block1437 : Inport  Port=3
  Block1438 : Constant  OutDataTypeStr=double  SampleTime=-1
  Block1439 : MinMax  Function=max  Inputs=2  RndMeth=Zero  SaturateOnIntegerOverflow=on  InputSameDT=on
  Block1440 : Product  Inputs=*/  OutDataTypeStr=double  SaturateOnIntegerOverflow=on  RndMeth=Zero  InputSameDT=on
      settings: AttributesFormatString=Attribute300
  Block1441 : Sum  Inputs=+-  OutDataTypeStr=double  RndMeth=Zero  SaturateOnIntegerOverflow=on  InputSameDT=on
  Block1442 : Sum  Inputs=2  OutDataTypeStr=double  RndMeth=Zero  SaturateOnIntegerOverflow=on  InputSameDT=on
  Block1443 : Outport
  wires:
  Block1435/1 -> Block1442/2, Block1441/2
  Block1436/1 -> Block1441/1
  Block1441/1 -> Block1440/1
  Block1440/1 -> Block1442/1
  Block1442/1 -> Block1443/1
  Block1438/1 -> Block1439/2
  Block1437/1 -> Block1439/1
  Block1439/1 -> Block1440/2

== system lib_all/SubSystem643/SubSystem645/SubSystem646 ==
  note: ***編集禁止***
  element : Inport
  Inside_DSR : DataStoreRead  DataStoreName=DSM77
  Selector : Selector  IndexOptions=Index vector (port)  Indices=[1 3]  OutputSizes=1
  Output : Outport
  wires:
  Inside_DSR/1 -> Selector/1
  element/1 -> Selector/2
  Selector/1 -> Output/1

== system lib_all/SubSystem643/SubSystem645/SubSystem647 ==
  note: ***編集禁止***
  element : Inport
  Inside_DSR : DataStoreRead  DataStoreName=DSM39
  Selector : Selector  IndexOptions=Index vector (port)  Indices=[1 3]  OutputSizes=1
  Output : Outport
  wires:
  Selector/1 -> Output/1
  element/1 -> Selector/2
  Inside_DSR/1 -> Selector/1

== system lib_all/SubSystem643/SubSystem645/SubSystem648 ==
  note: ***編集禁止***
  element : Inport
  Inside_DSR : DataStoreRead  DataStoreName=DSM51
  Selector : Selector  IndexOptions=Index vector (port)  Indices=[1 3]  OutputSizes=1
  Output : Outport
  wires:
  Inside_DSR/1 -> Selector/1
  element/1 -> Selector/2
  Selector/1 -> Output/1

== system lib_all/SubSystem643/SubSystem645/SubSystem649 ==
  note: ***編集禁止***
  element : Inport
  Inside_DSR : DataStoreRead  DataStoreName=DSM72
  Selector : Selector  IndexOptions=Index vector (port)  Indices=[1 3]  OutputSizes=1
  Output : Outport
  wires:
  Selector/1 -> Output/1
  element/1 -> Selector/2
  Inside_DSR/1 -> Selector/1

== system lib_all/SubSystem643/SubSystem645/SubSystem650 ==
  note: ***編集禁止***
  element : Inport
  Inside_DSR : DataStoreRead  DataStoreName=DSM53
  Selector : Selector  IndexOptions=Index vector (port)  Indices=[1 3]  OutputSizes=1
  Output : Outport
  wires:
  Inside_DSR/1 -> Selector/1
  element/1 -> Selector/2
  Selector/1 -> Output/1

== system lib_all/SubSystem643/SubSystem645/SubSystem651 ==
  note: ***編集禁止***
  element : Inport
  Inside_DSR : DataStoreRead  DataStoreName=DSM86
  Selector : Selector  IndexOptions=Index vector (port)  Indices=[1 3]  OutputSizes=1
  Output : Outport
  wires:
  Selector/1 -> Output/1
  element/1 -> Selector/2
  Inside_DSR/1 -> Selector/1

== system lib_all/SubSystem643/SubSystem645/SubSystem652 ==
  note: ***編集禁止***
  element : Inport
  Inside_DSR : DataStoreRead  DataStoreName=DSM62
  Selector : Selector  IndexOptions=Index vector (port)  Indices=[1 3]  OutputSizes=1
  Output : Outport
  wires:
  Inside_DSR/1 -> Selector/1
  element/1 -> Selector/2
  Selector/1 -> Output/1

== system lib_all/SubSystem643/SubSystem645/SubSystem653 ==
  note: ***編集禁止***
  element : Inport
  Inside_DSR : DataStoreRead  DataStoreName=DSM79
  Selector : Selector  IndexOptions=Index vector (port)  Indices=[1 3]  OutputSizes=1
  Output : Outport
  wires:
  Selector/1 -> Output/1
  element/1 -> Selector/2
  Inside_DSR/1 -> Selector/1

== system lib_all/SubSystem643/SubSystem645/SubSystem654 ==
  note: ***編集禁止***
  element : Inport
  Inside_DSR : DataStoreRead  DataStoreName=DSM81
  Selector : Selector  IndexOptions=Index vector (port)  Indices=[1 3]  OutputSizes=1
  Output : Outport
  wires:
  Inside_DSR/1 -> Selector/1
  element/1 -> Selector/2
  Selector/1 -> Output/1

== system lib_all/SubSystem643/SubSystem645/SubSystem655 ==
  note: ***編集禁止***
  element : Inport
  Inside_DSR : DataStoreRead  DataStoreName=DSM80
  Selector : Selector  IndexOptions=Index vector (port)  Indices=[1 3]  OutputSizes=1
  Output : Outport
  wires:
  Selector/1 -> Output/1
  element/1 -> Selector/2
  Inside_DSR/1 -> Selector/1

== system lib_all/SubSystem643/SubSystem645/SubSystem656 ==
  Signal_in : Ground
  Signal_out : Terminator
  dtc : DataTypeConversion  SaturateOnIntegerOverflow=on  RndMeth=Zero
  wires:
  Signal_in/1 -> dtc/1
  dtc/1 -> Signal_out/1

== system lib_all/SubSystem660/SubSystem673/SubSystem675 ==
  note: ***編集禁止***
  In1 : Inport
  In2 : Inport  Port=2
  RO1 : RelationalOperator  Operator===  InputSameDT=on
  single1 : DataTypeConversion  OutDataTypeStr=single  SaturateOnIntegerOverflow=on  RndMeth=Zero
  single2 : DataTypeConversion  OutDataTypeStr=single  SaturateOnIntegerOverflow=on  RndMeth=Zero
  Out1 : Outport
  wires:
  single2/1 -> RO1/2
  single1/1 -> RO1/1
  In1/1 -> single1/1
  In2/1 -> single2/1
  RO1/1 -> Out1/1

== system lib_all/SubSystem660/SubSystem673/SubSystem676 ==
  note: Text93
  Block1516 : Inport
  Block1517 : Inport  Port=2
  Block1518 : Constant  OutDataTypeStr=double  SampleTime=-1
  Block1519 : Constant  Value=2  OutDataTypeStr=double  SampleTime=-1
  Block1520 : Constant  Value=36  OutDataTypeStr=double  SampleTime=-1
  Block1521 : Constant  Value=0  OutDataTypeStr=double  SampleTime=-1
  Block1522 : Constant  Value=72  OutDataTypeStr=double  SampleTime=-1
  Block1523 : Constant  Value=0  OutDataTypeStr=double  SampleTime=-1
  Block1524 : Constant  Value=720  OutDataTypeStr=double  SampleTime=-1
  Block1525 : Gain  Gain=5  SaturateOnIntegerOverflow=on  OutDataTypeStr=Inherit: Same as input  ParamDataTypeStr=Inherit: Same as input
      settings: AttributesFormatString=Attribute322
  Block1526 : Logic  Operator=OR
  Block1527 : Sum  Inputs=+-  OutDataTypeStr=double  RndMeth=Zero  SaturateOnIntegerOverflow=on  InputSameDT=on
  Block1528 : Switch  Threshold=0.5  RndMeth=Zero  SaturateOnIntegerOverflow=on  InputSameDT=on
  SubSystem677 : Subsystem
  SubSystem678 : Subsystem
  SubSystem679 : Subsystem
      settings: RTWSystemCode=Inline
  SubSystem681 : Subsystem
      settings: RTWSystemCode=Inline
  Block1541 : Outport
  wires:
  Block1518/1 -> SubSystem677/1(In1)
  Block1519/1 -> SubSystem678/2(In2)
  Block1517/1 -> SubSystem677/2(In2), SubSystem678/1(In1)
  SubSystem677/1(Out1) -> Block1526/1
  SubSystem678/1(Out1) -> Block1526/2
  Block1516/1 -> Block1527/1, Block1528/3
  Block1520/1 -> Block1527/2
  Block1527/1 -> SubSystem681/2(Block1536)
  Block1521/1 -> SubSystem681/3(Block1537)
  Block1522/1 -> SubSystem681/1(Block1535)
  Block1526/1 -> Block1528/2
  SubSystem681/1(Block1540) -> Block1528/1
  SubSystem679/1(Block1534) -> Block1541/1
  Block1524/1 -> SubSystem679/1(Block1529)
  Block1528/1 -> Block1525/1
  Block1523/1 -> SubSystem679/3(Block1531)
  Block1525/1 -> SubSystem679/2(Block1530)

== system lib_all/SubSystem660/SubSystem673/SubSystem683 ==
  Block1542 : Inport
  Block1543 : EnablePort
  Block1544 : DataStoreWrite  DataStoreName=DSM217
  wires:
  Block1542/1 -> Block1544/1

== system lib_all/SubSystem660/SubSystem673/SubSystem684 ==
  note: Text95
  note: Text94
  Block1545 : Inport
  Block1546 : Inport  Port=2
  Block1547 : Constant  Value=360  OutDataTypeStr=double  SampleTime=-1
  Block1548 : Constant  Value=360  OutDataTypeStr=double  SampleTime=-1
  Block1549 : Sum  Inputs=+-  OutDataTypeStr=double  RndMeth=Zero  SaturateOnIntegerOverflow=on  InputSameDT=on
  Block1550 : Switch  Threshold=0.5  RndMeth=Zero  SaturateOnIntegerOverflow=on  InputSameDT=on
  Block1551 : Lookup2D  RowIndex=map_lib_02  ColumnIndex=map_lib_01  Table=map_lib_03  SaturateOnIntegerOverflow=on  InputSameDT=on
      settings: AttributesFormatString=<%<OutputValues>>\nAttribute325
  SubSystem685 : Subsystem
  Block1552 : Outport
  wires:
  Block1546/1 -> SubSystem685/1(In1), Block1550/3, Block1549/1
  Block1548/1 -> SubSystem685/2(In2)
  SubSystem685/1(Out1) -> Block1550/2
  Block1549/1 -> Block1550/1
  Block1547/1 -> Block1549/2
  Block1551/1 -> Block1552/1
  Block1545/1 -> Block1551/2
  Block1550/1 -> Block1551/1  "SigLabel37"
